simulink model "snap2_f_200msps_64i_4096c_for_8_11_2124fpg"  (R2019a)
settings: Solver=VariableStepDiscrete  StartTime=0.0  StopTime=10.0  RelTol=1e-3
undefined symbols (not defined in the file): datetime, posixtime
libraries: xbsIndex_r4 x17164, casper_library_misc x251, xps_library x14, casper_library_delays x12, casper_library_sources x4
(part 4 of 196)

== system snap2_f_200msps_64i_4096c_for_8_11_2124fpg/delay ==
  sync : Inport
  din : Inport  Port=2
  0 : Subsystem
  1 : Subsystem
  10 : Subsystem
  11 : Subsystem
  12 : Subsystem
  13 : Subsystem
  14 : Subsystem
  15 : Subsystem
  16 : Subsystem
  17 : Subsystem
  18 : Subsystem
  19 : Subsystem
  2 : Subsystem
  20 : Subsystem
  21 : Subsystem
  22 : Subsystem
  23 : Subsystem
  24 : Subsystem
  25 : Subsystem
  26 : Subsystem
  27 : Subsystem
  28 : Subsystem
  29 : Subsystem
  3 : Subsystem
  30 : Subsystem
  31 : Subsystem
  32 : Subsystem
  33 : Subsystem
  34 : Subsystem
  35 : Subsystem
  36 : Subsystem
  37 : Subsystem
  38 : Subsystem
  39 : Subsystem
  4 : Subsystem
  40 : Subsystem
  41 : Subsystem
  42 : Subsystem
  43 : Subsystem
  44 : Subsystem
  45 : Subsystem
  46 : Subsystem
  47 : Subsystem
  48 : Subsystem
  49 : Subsystem
  5 : Subsystem
  50 : Subsystem
  51 : Subsystem
  52 : Subsystem
  53 : Subsystem
  54 : Subsystem
  55 : Subsystem
  56 : Subsystem
  57 : Subsystem
  58 : Subsystem
  59 : Subsystem
  6 : Subsystem
  60 : Subsystem
  61 : Subsystem
  62 : Subsystem
  63 : Subsystem
  7 : Subsystem
  8 : Subsystem
  9 : Subsystem
  Constant : xbsIndex_r4/Constant  const=2^max_delay_bits  gui_display_data_type=Fixed-point  arith_type=Unsigned  n_bits=32  bin_pt=0  preci_type=Single  exp_width=8  frac_width=24  explicit_period=on  period=1  equ=P=C  opselect=C  inp2=PCIN>>17  opr=+  inp1=P  carry=CIN  dbl_ovrd=off  block_type=constant
  Delay : xbsIndex_r4/Delay  rst=off  infoeditControl=Selection of Reset will increase slice count due to use of real FFs and instead of SRLs  en=off  latency=7  reg_retiming=on  dbl_ovrd=off  block_type=delay
  bus_create : Subsystem  AncestorBlock=casper_library_flow_control/bus_create  mask(bus_create){inputNum=64}
      mask prompts: inputNum "Number of inputs:"
      mask init:
      | bus_create_init(gcb,...
      |     'inputNum', inputNum);
  bus_expand : Subsystem  AncestorBlock=casper_library_flow_control/bus_expand  mask(bus_expand){mode=divisions of equal size, outputNum=8*8, outputWidth=10, outputBinaryPt=0, outputArithmeticType=0, show_format=off, outputToWorkspace=off, variablePrefix=out, outputToModelAsWell=on}
      mask prompts: outputNum "Number of outputs:"; outputWidth "Output width:"; outputBinaryPt "Output binary point position:"; outputArithmeticType "Output arithmetic type (ufix=0, fix=1, bool=2):"; show_format "Print format string?"; outputToWorkspace "Output to workspace?"; variablePrefix "Variable name prefix:"; outputToModelAsWell "Output to model as well?"
      mask help:
      | Expands a 'bus' made using a bus_create or Xilinx
      | concat block.
      | The input will be divided into the specified number
      | of outputs with the specified arithmetic types.
      | Divisions are made from most significant bit.
      mask init: same as snap2_f_200msps_64i_4096c_for_8_11_2124fpg/autocorr/bus_expand
      settings: AttributesFormatString=64 outputs
      mask TypeOptions mode: divisions of equal size | divisions of arbitrary size
      mask Callback mode: bus_expand_callback();
      mask Callback outputWidth: bus_expand_callback();
      mask Callback outputToWorkspace: bus_expand_callback();
  max_delay : Subsystem  Tag=xps:sw_reg  AncestorBlock=xps_library/Memory/software_register  mask(swreg){io_dir=To Processor, io_delay=0, init_val=0, sample_period=1, names=reg, bitwidths=32, bin_pts=0, arith_types=0, sim_port=on, show_format=off, desc_str=|}
      mask desc_str=|
      | Interpretted as a unint32 value, contains the maximum
      | allowed delay, in ADC samples, which may be applied
      | to a data stream.
      mask prompts: io_dir "I/O direction"; io_delay "I/O delay"; init_val "Initial Value"; sample_period "Sample period"; names "Bitfield names [msb...lsb]"; bitwidths "Bitfield widths"; bin_pts "Bitfield binary pts"; arith_types "Bitfield types, ufix=0, fix=1, bool=2"; sim_port "Provide sim input/output?"; show_format "Print format string?"; desc_str "Description"
      mask help: A 32-bit software-accessible register. Can be divided into bitfields of varying widths and types using the fields in the block mask.
      mask init:
      | swreg_init(gcb)
      settings: AttributesFormatString=1output
      mask TypeOptions io_dir: To Processor | From Processor
      mask Callback io_dir:
      | mask_enables = get_param(gcb, 'MaskEnables');
      | mask_names = get_param(gcb, 'MaskNames');
      | if strcmp(get_param(gcb, 'io_dir'), 'To Processor'),
      |     mask_enables{ismember(mask_names, 'sample_period')} = 'off';
      | else
      |     mask_enables{ismember(mask_names, 'sample_period')} = 'on';
      | end
      | set_param(gcb, 'MaskEnables', mask_enables);
      mask DialogControl ParameterTabVar0 Prompt: Setup
  sync_out : Outport
  dout : Outport  Port=2
  wires:
  sync/1 -> Delay/1
  din/1 -> bus_expand/1(bus_in)
  bus_expand/1(msb_out64) -> 0/1(din)
  bus_create/1(bus_out) -> dout/1
  Constant/1 -> max_delay/1(out_reg)
  Delay/1 -> sync_out/1
  1/1(dout) -> bus_create/2(in2)
  0/1(dout) -> bus_create/1(in1)
  bus_expand/2(out63) -> 1/1(din)
  bus_expand/19(out46) -> 18/1(din)
  bus_expand/21(out44) -> 20/1(din)
  bus_expand/23(out42) -> 22/1(din)
  bus_expand/29(out36) -> 28/1(din)
  bus_expand/31(out34) -> 30/1(din)
  bus_expand/33(out32) -> 32/1(din)
  bus_expand/37(out28) -> 36/1(din)
  bus_expand/41(out24) -> 40/1(din)
  bus_expand/43(out22) -> 42/1(din)
  bus_expand/45(out20) -> 44/1(din)
  bus_expand/47(out18) -> 46/1(din)
  bus_expand/51(out14) -> 50/1(din)
  bus_expand/55(out10) -> 54/1(din)
  bus_expand/59(out6) -> 58/1(din)
  bus_expand/61(out4) -> 60/1(din)
  bus_expand/63(out2) -> 62/1(din)
  17/1(dout) -> bus_create/18(in18)
  3/1(dout) -> bus_create/4(in4)
  5/1(dout) -> bus_create/6(in6)
  7/1(dout) -> bus_create/8(in8)
  9/1(dout) -> bus_create/10(in10)
  11/1(dout) -> bus_create/12(in12)
  13/1(dout) -> bus_create/14(in14)
  15/1(dout) -> bus_create/16(in16)
  19/1(dout) -> bus_create/20(in20)
  21/1(dout) -> bus_create/22(in22)
  23/1(dout) -> bus_create/24(in24)
  25/1(dout) -> bus_create/26(in26)
  27/1(dout) -> bus_create/28(in28)
  29/1(dout) -> bus_create/30(in30)
  31/1(dout) -> bus_create/32(in32)
  33/1(dout) -> bus_create/34(in34)
  35/1(dout) -> bus_create/36(in36)
  37/1(dout) -> bus_create/38(in38)
  39/1(dout) -> bus_create/40(in40)
  41/1(dout) -> bus_create/42(in42)
  43/1(dout) -> bus_create/44(in44)
  45/1(dout) -> bus_create/46(in46)
  47/1(dout) -> bus_create/48(in48)
  49/1(dout) -> bus_create/50(in50)
  51/1(dout) -> bus_create/52(in52)
  53/1(dout) -> bus_create/54(in54)
  55/1(dout) -> bus_create/56(in56)
  57/1(dout) -> bus_create/58(in58)
  59/1(dout) -> bus_create/60(in60)
  61/1(dout) -> bus_create/62(in62)
  63/1(dout) -> bus_create/64(in64)
  16/1(dout) -> bus_create/17(in17)
  2/1(dout) -> bus_create/3(in3)
  4/1(dout) -> bus_create/5(in5)
  6/1(dout) -> bus_create/7(in7)
  8/1(dout) -> bus_create/9(in9)
  10/1(dout) -> bus_create/11(in11)
  12/1(dout) -> bus_create/13(in13)
  14/1(dout) -> bus_create/15(in15)
  18/1(dout) -> bus_create/19(in19)
  20/1(dout) -> bus_create/21(in21)
  22/1(dout) -> bus_create/23(in23)
  24/1(dout) -> bus_create/25(in25)
  26/1(dout) -> bus_create/27(in27)
  28/1(dout) -> bus_create/29(in29)
  30/1(dout) -> bus_create/31(in31)
  32/1(dout) -> bus_create/33(in33)
  34/1(dout) -> bus_create/35(in35)
  36/1(dout) -> bus_create/37(in37)
  38/1(dout) -> bus_create/39(in39)
  40/1(dout) -> bus_create/41(in41)
  42/1(dout) -> bus_create/43(in43)
  44/1(dout) -> bus_create/45(in45)
  46/1(dout) -> bus_create/47(in47)
  48/1(dout) -> bus_create/49(in49)
  50/1(dout) -> bus_create/51(in51)
  52/1(dout) -> bus_create/53(in53)
  54/1(dout) -> bus_create/55(in55)
  56/1(dout) -> bus_create/57(in57)
  58/1(dout) -> bus_create/59(in59)
  60/1(dout) -> bus_create/61(in61)
  62/1(dout) -> bus_create/63(in63)
  bus_expand/3(out62) -> 2/1(din)
  bus_expand/5(out60) -> 4/1(din)
  bus_expand/7(out58) -> 6/1(din)
  bus_expand/9(out56) -> 8/1(din)
  bus_expand/11(out54) -> 10/1(din)
  bus_expand/13(out52) -> 12/1(din)
  bus_expand/15(out50) -> 14/1(din)
  bus_expand/25(out40) -> 24/1(din)
  bus_expand/27(out38) -> 26/1(din)
  bus_expand/35(out30) -> 34/1(din)
  bus_expand/39(out26) -> 38/1(din)
  bus_expand/49(out16) -> 48/1(din)
  bus_expand/53(out12) -> 52/1(din)
  bus_expand/57(out8) -> 56/1(din)
  bus_expand/17(out48) -> 16/1(din)
  bus_expand/4(out61) -> 3/1(din)
  bus_expand/6(out59) -> 5/1(din)
  bus_expand/8(out57) -> 7/1(din)
  bus_expand/10(out55) -> 9/1(din)
  bus_expand/12(out53) -> 11/1(din)
  bus_expand/14(out51) -> 13/1(din)
  bus_expand/16(out49) -> 15/1(din)
  bus_expand/20(out45) -> 19/1(din)
  bus_expand/22(out43) -> 21/1(din)
  bus_expand/24(out41) -> 23/1(din)
  bus_expand/26(out39) -> 25/1(din)
  bus_expand/28(out37) -> 27/1(din)
  bus_expand/30(out35) -> 29/1(din)
  bus_expand/32(out33) -> 31/1(din)
  bus_expand/34(out31) -> 33/1(din)
  bus_expand/36(out29) -> 35/1(din)
  bus_expand/38(out27) -> 37/1(din)
  bus_expand/40(out25) -> 39/1(din)
  bus_expand/42(out23) -> 41/1(din)
  bus_expand/44(out21) -> 43/1(din)
  bus_expand/46(out19) -> 45/1(din)
  bus_expand/48(out17) -> 47/1(din)
  bus_expand/50(out15) -> 49/1(din)
  bus_expand/52(out13) -> 51/1(din)
  bus_expand/54(out11) -> 53/1(din)
  bus_expand/56(out9) -> 55/1(din)
  bus_expand/58(out7) -> 57/1(din)
  bus_expand/60(out5) -> 59/1(din)
  bus_expand/62(out3) -> 61/1(din)
  bus_expand/64(lsb_out1) -> 63/1(din)
  bus_expand/18(out47) -> 17/1(din)

== system snap2_f_200msps_64i_4096c_for_8_11_2124fpg/eq ==
  sync : Inport
  din : Inport  Port=2
  bus_create2 : Subsystem  AncestorBlock=casper_library_flow_control/bus_create  mask(bus_create){inputNum=4}
      mask prompts: inputNum "Number of inputs:"
      mask init:
      | bus_create_init(gcb,...
      |     'inputNum', inputNum);
  bus_expand4 : Subsystem  AncestorBlock=casper_library_flow_control/bus_expand  mask(bus_expand){mode=divisions of equal size, outputNum=4, outputWidth=36*8, outputBinaryPt=0, outputArithmeticType=0, show_format=off, outputToWorkspace=off, variablePrefix=out, outputToModelAsWell=on}
      mask prompts: outputNum "Number of outputs:"; outputWidth "Output width:"; outputBinaryPt "Output binary point position:"; outputArithmeticType "Output arithmetic type (ufix=0, fix=1, bool=2):"; show_format "Print format string?"; outputToWorkspace "Output to workspace?"; variablePrefix "Variable name prefix:"; outputToModelAsWell "Output to model as well?"
      mask help:
      | Expands a 'bus' made using a bus_create or Xilinx
      | concat block.
      | The input will be divided into the specified number
      | of outputs with the specified arithmetic types.
      | Divisions are made from most significant bit.
      mask init: same as snap2_f_200msps_64i_4096c_for_8_11_2124fpg/autocorr/bus_expand
      settings: AttributesFormatString=4 outputs
      mask TypeOptions mode: divisions of equal size | divisions of arbitrary size
      mask Callback mode: bus_expand_callback();
      mask Callback outputWidth: bus_expand_callback();
      mask Callback outputToWorkspace: bus_expand_callback();
  core0 : Subsystem  mask{nchan=12-3, nants=16}
      mask prompts: nchan "Number of fft channels (2^?)"; nants "Number of Antennas"
  core1 : Subsystem  mask{nchan=12-3, nants=16}
      mask prompts: nchan "Number of fft channels (2^?)"; nants "Number of Antennas"
  core2 : Subsystem  mask{nchan=12-3, nants=16}
      mask prompts: nchan "Number of fft channels (2^?)"; nants "Number of Antennas"
  core3 : Subsystem  mask{nchan=12-3, nants=16}
      mask prompts: nchan "Number of fft channels (2^?)"; nants "Number of Antennas"
  sync_out : Outport
  dout : Outport  Port=2
  wires:
  core0/1(sync_out) -> sync_out/1
  din/1 -> bus_expand4/1(bus_in)
  sync/1 -> [sync_in]
  [sync_in] -> core0/1(sync)
  [sync_in] -> core1/1(sync)
  [sync_in] -> core2/1(sync)
  [sync_in] -> core3/1(sync)
  bus_expand4/1(msb_out4) -> core0/2(in)
  bus_expand4/2(out3) -> core1/2(in)
  bus_expand4/3(out2) -> core2/2(in)
  bus_expand4/4(lsb_out1) -> core3/2(in)
  core1/1(sync_out) -> ?
  core2/1(sync_out) -> ?
  core3/1(sync_out) -> ?
  core1/2(dout) -> bus_create2/2(in2)
  core2/2(dout) -> bus_create2/3(in3)
  core0/2(dout) -> bus_create2/1(in1)
  core3/2(dout) -> bus_create2/4(in4)
  bus_create2/1(bus_out) -> dout/1

== system snap2_f_200msps_64i_4096c_for_8_11_2124fpg/eth ==
  note: bit 19
  note: bit 18
  note: bit 1
  note: bit 0
  note: only allow enable
      | state to change at the end
      | of a packet.
      |
      | This avoids overflows arising
      | from part of a packet being
      | clocked in to the core
      | but the EOF being disable
  data : Inport
  vld : Inport  Port=2
  eof : Inport  Port=3
  dest_ip : Inport  Port=4
  port : Inport  Port=5
  Constant : xbsIndex_r4/Constant  const=0  gui_display_data_type=Boolean  arith_type=Boolean  n_bits=1  bin_pt=0  preci_type=Single  exp_width=8  frac_width=24  explicit_period=on  period=1  equ=P=C  opselect=C  inp2=PCIN>>17  opr=+  inp1=P  carry=CIN  dbl_ovrd=off  block_type=constant
  Constant1 : xbsIndex_r4/Constant  const=0  gui_display_data_type=Boolean  arith_type=Boolean  n_bits=1  bin_pt=0  preci_type=Single  exp_width=8  frac_width=24  explicit_period=on  period=1  equ=P=C  opselect=C  inp2=PCIN>>17  opr=+  inp1=P  carry=CIN  dbl_ovrd=off  block_type=constant
  Delay{,1..4} (5 alike) : xbsIndex_r4/Delay  rst=off  infoeditControl=Selection of Reset will increase slice count due to use of real FFs and instead of SRLs  en=off  latency=1  reg_retiming=off  dbl_ovrd=off  block_type=delay
  Logical : xbsIndex_r4/Logical  logical_function=AND  inputs=2  en=off  latency=0  precision=Full  arith_type=Unsigned  n_bits=16  bin_pt=0  align_bp=on  dbl_ovrd=off  block_type=logical
  Logical1 : xbsIndex_r4/Logical  logical_function=OR  inputs=2  en=off  latency=0  precision=Full  arith_type=Unsigned  n_bits=16  bin_pt=0  align_bp=on  dbl_ovrd=off  block_type=logical
  Register : xbsIndex_r4/Register  init=0  rst=off  en=on  dbl_ovrd=off  block_type=register
  Slice : xbsIndex_r4/Slice  nbits=1  boolean_output=on  mode=Lower Bit Location + Width  bit1=0  base1=MSB of Input  bit0=0  base0=LSB of Input  dbl_ovrd=off  block_type=slice
  Slice1 : xbsIndex_r4/Slice  nbits=1  boolean_output=on  mode=Lower Bit Location + Width  bit1=0  base1=MSB of Input  bit0=1  base0=LSB of Input  dbl_ovrd=off  block_type=slice
  Slice3 : xbsIndex_r4/Slice  nbits=1  boolean_output=on  mode=Lower Bit Location + Width  bit1=0  base1=MSB of Input  bit0=2+16  base0=LSB of Input  dbl_ovrd=off  block_type=slice
  Slice4 : xbsIndex_r4/Slice  nbits=1  boolean_output=on  mode=Lower Bit Location + Width  bit1=0  base1=MSB of Input  bit0=3+16  base0=LSB of Input  dbl_ovrd=off  block_type=slice
  ctrl : Subsystem  Tag=xps:sw_reg  AncestorBlock=xps_library/Memory/software_register  mask(swreg){io_dir=From Processor, io_delay=0, init_val=0, sample_period=1, names=reg, bitwidths=32, bin_pts=0, arith_types=0, sim_port=on, show_format=off, desc_str=|}
      mask desc_str=|
      | 40GbE control register. Bit ``0`` is an active high reset
      | of the 40GbE interface core. Bit ``1`` is an active
      | high transmission enable for the core, which takes
      | effect either after the next packet is sent, or when
      | the ``force`` flag is asserted. Bit ``18`` is
      | an active high reset for statistics provided by the
      | Ethernet core. Bit ``19`` is an active high ``force``
      | signal, which causes the current transmission enable
      | flag to immediately take effect.
      mask prompts: io_dir "I/O direction"; io_delay "I/O delay"; init_val "Initial Value"; sample_period "Sample period"; names "Bitfield names [msb...lsb]"; bitwidths "Bitfield widths"; bin_pts "Bitfield binary pts"; arith_types "Bitfield types, ufix=0, fix=1, bool=2"; sim_port "Provide sim input/output?"; show_format "Print format string?"; desc_str "Description"
      mask help: A 32-bit software-accessible register. Can be divided into bitfields of varying widths and types using the fields in the block mask.
      mask init:
      | swreg_init(gcb)
      settings: AttributesFormatString=1input
      mask TypeOptions io_dir: To Processor | From Processor
      mask Callback io_dir:
      | mask_enables = get_param(gcb, 'MaskEnables');
      | mask_names = get_param(gcb, 'MaskNames');
      | if strcmp(get_param(gcb, 'io_dir'), 'To Processor'),
      |     mask_enables{ismember(mask_names, 'sample_period')} = 'off';
      | else
      |     mask_enables{ismember(mask_names, 'sample_period')} = 'on';
      | end
      | set_param(gcb, 'MaskEnables', mask_enables);
      mask DialogControl ParameterTabVar0 Prompt: Setup
  forty_gbe : Subsystem  Tag=xps:forty_gbe  AncestorBlock=xps_library/IO/forty_gbe  InitFcn=forty_gbe_init  mask(forty_gbe){flavour=cx4, slot=1, port_r1=0, port_r2_cx4=0, port_r2_sfpp=0, port=0, rx_dist_ram=on, large_frames=on, input_pipeline_delay=0, show_param=on, pre_emph_r2=0.74, pre_emph=3, post_emph_r2=0.18, rxeqmix_r2=7, swing_r2=940, swing=800, fab_en=on, fab_mac=hex2dec('123456780000'), fab_ip=192*(2^24) + 168*(2^16) + 5*(2^8) + 20*(2^0), fab_udp=10000, fab_gate=1, cpu_rx_en=off, cpu_tx_en=off, ttl=hex2dec('FF'), promisc_mode=off, debug_ctr_width=32, debug_en_all=off, debug_dis_all=off, txctr=on, txerrctr=off, txerrctr_len=100, txofctr=on, txfullctr=on, txvldctr=on, txsnaplen=0 - no snap, rxctr=off, rxerrctr=off, rxerrctr_len=100, rxofctr=off, rxbadctr=off, rxvldctr=off, rxeofctr=off, rxsnaplen=0 - no snap, rxsrcip=off, rxdstip=off}
      mask prompts: flavour "Mezzanine card flavour"; slot "Card slot"; port_r1 "Port"; port_r2_cx4 "Port"; port_r2_sfpp "Port"; rx_dist_ram "Shallow RX Fifo (Beware overruns!)"; large_frames "Enable Large TX Frames (8k+512)"; input_pipeline_delay "Incoming pipeline delay"; show_param "---------------------- Show Implementation Parameters -----------------------------------------------------------------------------------"; pre_emph_r2 "Pre-emphasis (dB)"; pre_emph "Pre-emphasis"; post_emph_r2 "Post-emphasis (dB)"; rxeqmix_r2 "Receive equalisation"; swing_r2 "Driver Swing Control (mVppd)"; swing "Differential swing"; fab_en "Enable fabric on startup"; fab_mac "Fabric MAC Address"; fab_ip "Fabric IP Address"; fab_udp "Fabric UDP Port"; fab_gate "Fabric Gateway"; cpu_rx_en "Enable CPU RX"; cpu_tx_en "Enable CPU TX"; promisc_mode "Promiscuous mode"; debug_ctr_width "Debug counter width"; debug_en_all "Enable all counters (overrides individual settings)"; debug_dis_all "Disable all counters (overrides individual settings)"; txctr "TX - core_txctr"; txerrctr "TX packet length error - core_txerrctr"; txerrctr_len "-- expected TX packet length"; txofctr "TX overflow - core_txofctr"; txfullctr "TX full - core_txfullctr"; txvldctr "TX valid cycle - core_txvldctr"; txsnaplen "TX snapshot length"; rxctr "RX - core_rxctr"; rxerrctr "RX packet length error - core_rxerrctr"; rxerrctr_len "-- expected RX packet length"; rxofctr "RX overflow - core_rxofctr"; rxbadctr "RX bad - core_rxbadctr"; rxvldctr "RX valid cycle - core_rxvldctr"; rxeofctr "RX eof - core_rxeofctr"; rxsnaplen "RX snapshot length"; rxsrcip "RX snapshot - include source IPs"; rxdstip "RX snapshot - include destination IPs"
      mask help: This block sends and receives UDP frames (packets). It accepts a 256 bit wide data stream with user-determined frame breaks. The data stream is wrapped in a UDP frame for transmission. Incoming UDP packets are unwrapped and the data presented as a 256 bit wide stream.
      mask init:
      | temp = eval(get_param(gcb, 'debug_ctr_width'));
      | if temp <= 0,
      |     error('Debug counters cannot be <= zero.');
      | end
      | forty_gbe_mask(gcb)
      settings: AttributesFormatString=incoming_latency=0
      mask TypeOptions flavour: sfp+ | cx4
      mask Callback flavour: forty_gbe_callback
      mask TypeOptions slot: 0 | 1
      mask TypeOptions port_r1: 0 | 1 | 2 | 3
      mask TypeOptions port_r2_cx4: 0 | 1 | 2
      mask TypeOptions port_r2_sfpp: 0 | 1 | 2 | 3
      mask TypeOptions port: 0 | 1 | 2 | 3
      mask Callback show_param: forty_gbe_callback
      mask TypeOptions pre_emph_r2: 0.15 | 0.3 | 0.45 | 0.61 | 0.74 | 0.91 | 1.07 | 1.25 | 1.36 | 1.55 | 1.74 | 1.94 | 2.11 | 2.32 | 2.54 | 2.77
      mask TypeOptions pre_emph: 0 | 1 | 2 | 3
      mask TypeOptions post_emph_r2: 0.18 | 0.19 | 0.2 | 0.39 | 0.63 | 0.82 | 1.07 | 1.32 | 1.6 | 1.65 | 1.94 | 2.21 | 2.52 | 2.76 | 3.08 | 3.41 | 3.77 | 3.97 | 4.36 | 4.73 | 5.16 | 5.47 | 5.93 | 6.38 | 6.89
      mask TypeOptions rxeqmix_r2: 0 | 1 | 2 | 3 | 4 | 5 | 6 | 7
      mask TypeOptions swing_r2: 110 | 210 | 310 | 400 | 480 | 570 | 660 | 740 | 810 | 880 | 940 | 990 | 1040 | 1080 | 1110 | 1130
      mask TypeOptions swing: 400 | 500 | 600 | 700 | 800
      mask Callback debug_en_all:
      | if strcmp(get_param(gcb, 'debug_en_all'), 'on')
      |     set_param(gcb, 'debug_dis_all', 'off')
      | end
      mask Callback debug_dis_all:
      | if strcmp(get_param(gcb, 'debug_dis_all'), 'on')
      |     set_param(gcb, 'debug_en_all', 'off')
      | end
      mask Callback txerrctr:
      | blk = gcb;
      | mask_visibilities = get_param(blk, 'MaskVisibilities');
      | mask_names = get_param(blk, 'MaskNames');
      | if strcmp(get_param(blk, 'txerrctr'), 'on')
      |     mask_visibilities{ismember(mask_names, 'txerrctr_len')} = 'on';
      | else
      |     mask_visibilities{ismember(mask_names, 'txerrctr_len')} = 'off';
      | end
      | set_param(blk, 'MaskVisibilities', mask_visibilities);
      mask TypeOptions txsnaplen: 0 - no snap | 256 | 512 | 1024 | 2048 | 4096 | 8192 | 16384
      mask Callback rxerrctr:
      | blk = gcb;
      | mask_visibilities = get_param(blk, 'MaskVisibilities');
      | mask_names = get_param(blk, 'MaskNames');
      | if strcmp(get_param(blk, 'rxerrctr'), 'on')
      |     mask_visibilities{ismember(mask_names, 'rxerrctr_len')} = 'on';
      | else
      |     mask_visibilities{ismember(mask_names, 'rxerrctr_len')} = 'off';
      | end
      | set_param(blk, 'MaskVisibilities', mask_visibilities);
      mask TypeOptions rxsnaplen: 0 - no snap | 256 | 512 | 1024 | 2048 | 4096 | 8192 | 16384
      mask DialogControl ParameterTabVar0 Prompt: Core
      mask DialogControl ParameterTabVar01 Prompt: Debug counters
      mask DialogControl Control2 Prompt: -------------------------------------
      mask DialogControl Control2_copy Prompt: -------------------------------------
  wires:
  port/1 -> Delay3/1
  forty_gbe/12(rx_overrun) -> [gbe_sw_rx_orun]
  [gbe_sw_rst] -> forty_gbe/1(rst)
  data/1 -> Delay/1
  eof/1 -> Logical1/2, Delay4/1
  dest_ip/1 -> Delay2/1
  Logical/1 -> Delay1/1
  vld/1 -> Logical/1
  ctrl/1(in_reg) -> Slice4/1, Slice3/1, Slice1/1, Slice/1
  Slice/1 -> [gbe_sw_rst]
  Slice1/1 -> [gbe_sw_en]
  Slice3/1 -> [gbe_stat_rst]
  [gbe_stat_rst] -> forty_gbe/9(debug_rst)
  forty_gbe/5(tx_overflow) -> [gbe_sw_tx_oflow]
  forty_gbe/4(tx_afull) -> [gbe_sw_tx_afull]
  forty_gbe/3(led_tx) -> [gbe_sw_led_tx]
  forty_gbe/2(led_rx) -> [gbe_sw_led_rx]
  forty_gbe/1(led_up) -> [gbe_sw_led_up]
  forty_gbe/11(rx_bad_frame) -> [gbe_sw_rx_bfr]
  Constant/1 -> forty_gbe/7(rx_ack)
  [gbe_sw_en] -> Register/1
  Register/1 -> Logical/2
  Slice4/1 -> [force_en]
  Logical1/1 -> Register/2
  [force_en] -> Logical1/1
  Delay/1 -> forty_gbe/2(tx_data)
  Delay1/1 -> forty_gbe/3(tx_valid)
  Delay2/1 -> forty_gbe/4(tx_dest_ip)
  Delay3/1 -> forty_gbe/5(tx_dest_port)
  Delay4/1 -> forty_gbe/6(tx_end_of_frame)
  Constant1/1 -> forty_gbe/8(rx_overrun_ack)

== system snap2_f_200msps_64i_4096c_for_8_11_2124fpg/input ==
  adc : Inport
  noise : Inport  Port=2
  sync : Inport  Port=3
  Constant4 : xbsIndex_r4/Constant  const=0  gui_display_data_type=Fixed-point  arith_type=Unsigned  n_bits=10*64  bin_pt=0  preci_type=Single  exp_width=8  frac_width=24  explicit_period=on  period=1  equ=P=C  opselect=C  inp2=PCIN>>17  opr=+  inp1=P  carry=CIN  dbl_ovrd=off  block_type=constant
  bit_stats : Subsystem
  bus_create2 : Subsystem  AncestorBlock=casper_library_flow_control/bus_create  mask(bus_create){inputNum=4}
      mask prompts: inputNum "Number of inputs:"
      mask init:
      | bus_create_init(gcb,...
      |     'inputNum', inputNum);
  bus_mux : Subsystem  AncestorBlock=casper_library_bus/bus_mux  mask(bus_mux){n_inputs=3, n_bits=10 * ones(64,1), cmplx=off, misc=on, mux_latency=1}
      mask prompts: n_inputs "number of inputs"; n_bits "input bit widths"; cmplx "complex data"; misc "misc support"; mux_latency "mux latency"
      mask help: Mux components of bus
      mask init:
      | bus_mux_init(gcb, ...
      |     'n_inputs', n_inputs, ...
      |     'n_bits', n_bits, ...
      |     'cmplx', cmplx, ...
      |     'misc', misc, ...
      |     'mux_latency', mux_latency);
  rms : Subsystem
  slice3 : xbsIndex_r4/Slice  nbits=2*64  boolean_output=off  mode=Lower Bit Location + Width  bit1=0  base1=MSB of Input  bit0=0  base0=LSB of Input  dbl_ovrd=off  block_type=slice
  source_sel0 : Subsystem  Tag=xps:sw_reg  AncestorBlock=xps_library/Memory/software_register  mask(swreg){io_dir=From Processor, io_delay=0, init_val=0, sample_period=1, names=reg, bitwidths=32, bin_pts=0, arith_types=0, sim_port=on, show_format=off, desc_str=|}
      mask desc_str=|
      | Selector control to determine if signal streams carry
      | ADC samples, digital noise samples, or zeros.
      | Streams ``n=0..15`` is controlled by register
      | bits ``[2n+1:2n]``. Value ``0`` selects noise;
      | ``1`` selects ADC; ``2`` selects zeros.
      mask prompts: io_dir "I/O direction"; io_delay "I/O delay"; init_val "Initial Value"; sample_period "Sample period"; names "Bitfield names [msb...lsb]"; bitwidths "Bitfield widths"; bin_pts "Bitfield binary pts"; arith_types "Bitfield types, ufix=0, fix=1, bool=2"; sim_port "Provide sim input/output?"; show_format "Print format string?"; desc_str "Description"
      mask help: A 32-bit software-accessible register. Can be divided into bitfields of varying widths and types using the fields in the block mask.
      mask init:
      | swreg_init(gcb)
      settings: AttributesFormatString=1input
      mask TypeOptions io_dir: To Processor | From Processor
      mask Callback io_dir:
      | mask_enables = get_param(gcb, 'MaskEnables');
      | mask_names = get_param(gcb, 'MaskNames');
      | if strcmp(get_param(gcb, 'io_dir'), 'To Processor'),
      |     mask_enables{ismember(mask_names, 'sample_period')} = 'off';
      | else
      |     mask_enables{ismember(mask_names, 'sample_period')} = 'on';
      | end
      | set_param(gcb, 'MaskEnables', mask_enables);
      mask DialogControl ParameterTabVar0 Prompt: Setup
  source_sel1 : Subsystem  Tag=xps:sw_reg  AncestorBlock=xps_library/Memory/software_register  mask(swreg){io_dir=From Processor, io_delay=0, init_val=0, sample_period=1, names=reg, bitwidths=32, bin_pts=0, arith_types=0, sim_port=on, show_format=off, desc_str=|}
      mask desc_str=|
      | Selector control to determine if signal streams carry
      | ADC samples, digital noise samples, or zeros.
      | Streams ``n=16..31`` is controlled by register
      | bits ``[2(n-16)+1:2(n-16)]``. Value ``0`` selects noise;
      | ``1`` selects ADC; ``2`` selects zeros.
      mask prompts: io_dir "I/O direction"; io_delay "I/O delay"; init_val "Initial Value"; sample_period "Sample period"; names "Bitfield names [msb...lsb]"; bitwidths "Bitfield widths"; bin_pts "Bitfield binary pts"; arith_types "Bitfield types, ufix=0, fix=1, bool=2"; sim_port "Provide sim input/output?"; show_format "Print format string?"; desc_str "Description"
      mask help: A 32-bit software-accessible register. Can be divided into bitfields of varying widths and types using the fields in the block mask.
      mask init:
      | swreg_init(gcb)
      settings: AttributesFormatString=1input
      mask TypeOptions io_dir: To Processor | From Processor
      mask Callback io_dir:
      | mask_enables = get_param(gcb, 'MaskEnables');
      | mask_names = get_param(gcb, 'MaskNames');
      | if strcmp(get_param(gcb, 'io_dir'), 'To Processor'),
      |     mask_enables{ismember(mask_names, 'sample_period')} = 'off';
      | else
      |     mask_enables{ismember(mask_names, 'sample_period')} = 'on';
      | end
      | set_param(gcb, 'MaskEnables', mask_enables);
      mask DialogControl ParameterTabVar0 Prompt: Setup
  source_sel2 : Subsystem  Tag=xps:sw_reg  AncestorBlock=xps_library/Memory/software_register  mask(swreg){io_dir=From Processor, io_delay=0, init_val=0, sample_period=1, names=reg, bitwidths=32, bin_pts=0, arith_types=0, sim_port=on, show_format=off, desc_str=|}
      mask desc_str=|
      | Selector control to determine if signal streams carry
      | ADC samples, digital noise samples, or zeros.
      | Streams ``n=32..47`` is controlled by register
      | bits ``[2(n-32)+1:2(n-32)]``. Value ``0`` selects noise;
      | ``1`` selects ADC; ``2`` selects zeros.
      mask prompts: io_dir "I/O direction"; io_delay "I/O delay"; init_val "Initial Value"; sample_period "Sample period"; names "Bitfield names [msb...lsb]"; bitwidths "Bitfield widths"; bin_pts "Bitfield binary pts"; arith_types "Bitfield types, ufix=0, fix=1, bool=2"; sim_port "Provide sim input/output?"; show_format "Print format string?"; desc_str "Description"
      mask help: A 32-bit software-accessible register. Can be divided into bitfields of varying widths and types using the fields in the block mask.
      mask init:
      | swreg_init(gcb)
      settings: AttributesFormatString=1input
      mask TypeOptions io_dir: To Processor | From Processor
      mask Callback io_dir:
      | mask_enables = get_param(gcb, 'MaskEnables');
      | mask_names = get_param(gcb, 'MaskNames');
      | if strcmp(get_param(gcb, 'io_dir'), 'To Processor'),
      |     mask_enables{ismember(mask_names, 'sample_period')} = 'off';
      | else
      |     mask_enables{ismember(mask_names, 'sample_period')} = 'on';
      | end
      | set_param(gcb, 'MaskEnables', mask_enables);
      mask DialogControl ParameterTabVar0 Prompt: Setup
  source_sel3 : Subsystem  Tag=xps:sw_reg  AncestorBlock=xps_library/Memory/software_register  mask(swreg){io_dir=From Processor, io_delay=0, init_val=0, sample_period=1, names=reg, bitwidths=32, bin_pts=0, arith_types=0, sim_port=on, show_format=off, desc_str=|}
      mask desc_str=|
      | Selector control to determine if signal streams carry
      | ADC samples, digital noise samples, or zeros.
      | Streams ``n=48..63`` is controlled by register
      | bits ``[2(n-48)+1:2(n-48)]``. Value ``0`` selects noise;
      | ``1`` selects ADC; ``2`` selects zeros.
      mask prompts: io_dir "I/O direction"; io_delay "I/O delay"; init_val "Initial Value"; sample_period "Sample period"; names "Bitfield names [msb...lsb]"; bitwidths "Bitfield widths"; bin_pts "Bitfield binary pts"; arith_types "Bitfield types, ufix=0, fix=1, bool=2"; sim_port "Provide sim input/output?"; show_format "Print format string?"; desc_str "Description"
      mask help: A 32-bit software-accessible register. Can be divided into bitfields of varying widths and types using the fields in the block mask.
      mask init:
      | swreg_init(gcb)
      settings: AttributesFormatString=1input
      mask TypeOptions io_dir: To Processor | From Processor
      mask Callback io_dir:
      | mask_enables = get_param(gcb, 'MaskEnables');
      | mask_names = get_param(gcb, 'MaskNames');
      | if strcmp(get_param(gcb, 'io_dir'), 'To Processor'),
      |     mask_enables{ismember(mask_names, 'sample_period')} = 'off';
      | else
      |     mask_enables{ismember(mask_names, 'sample_period')} = 'on';
      | end
      | set_param(gcb, 'MaskEnables', mask_enables);
      mask DialogControl ParameterTabVar0 Prompt: Setup
  sync_out : Outport
  dout : Outport  Port=2
  wires:
  Constant4/1 -> bus_mux/4(d2)
  sync/1 -> bus_mux/5(misci)
  bus_mux/1(out) -> dout/1, bit_stats/1(din), rms/1(in)
  slice3/1 -> bus_mux/1(sel)
  adc/1 -> bus_mux/3(d1)
  noise/1 -> bus_mux/2(d0)
  bus_mux/2(misco) -> sync_out/1
  source_sel1/1(in_reg) -> bus_create2/2(in2)
  source_sel0/1(in_reg) -> bus_create2/1(in1)
  source_sel2/1(in_reg) -> bus_create2/3(in3)
  bus_create2/1(bus_out) -> slice3/1
  source_sel3/1(in_reg) -> bus_create2/4(in4)

== system snap2_f_200msps_64i_4096c_for_8_11_2124fpg/noise ==
  sync_in : Inport
  arm_noise : Inport  Port=2
  BitBasher : xbsIndex_r4/BitBasher  bitexpr=|  display_expr=off  sr_1=1  arith_type1=Unsigned  bin_pt1=0  sr_2=2  arith_type2=Unsigned  bin_pt2=0  sr_3=3  arith_type3=Unsigned  bin_pt3=0  sr_4=4  arith_type4=Unsigned  bin_pt4=0  dbl_ovrd=off  block_type=bitbasher
      | <!DOCTYPE HTML PUBLIC "-//W3C//DTD HTML 4.0//EN" "http://www.w3.org/TR/REC-html40/strict.dtd">
      | <html><head><meta name="qrichtext" content="1" /><style type="text/css">
      | p, li { white-space: pre-wrap; }
      | </style></head><body style=" font-family:'Sans'; font-size:10pt; font-weight:400; font-style:normal;">
      | <p style=" margin-top:12px; margin-bottom:12px; margin-left:0px; margin-right:0px; -qt-block-indent:0; text-indent:0px;"><span style=" font-family:'MS Shell Dlg'; font-size:8pt;">s0={8'h5c, s32[7:0], s32[0:7], 8'ha3}<br />s1={8'h5c, s32[15:8], s32[8:15], 8'ha3}<br />s2={8'h5c, s32[23:16], s32[16:23], 8'ha3}<br />s3={8'h5c, s32[31:24], s32[24:31], 8'ha3}</span></p></body></html>
  Constant : Constant  Value=0
  Delay{,1..7} (8 alike) : xbsIndex_r4/Delay  rst=off  infoeditControl=Selection of Reset will increase slice count due to use of real FFs and instead of SRLs  en=off  latency=1  reg_retiming=off  dbl_ovrd=off  block_type=delay
  armed_trigger : casper_library_misc/armed_trigger
      settings: SourceProductName=CASPER DSP Blockset
  bus_create1 : Subsystem  AncestorBlock=casper_library_flow_control/bus_create  mask(bus_create){inputNum=8}
      mask prompts: inputNum "Number of inputs:"
      mask init:
      | bus_create_init(gcb,...
      |     'inputNum', inputNum);
  bus_create2 : Subsystem  AncestorBlock=casper_library_flow_control/bus_create  mask(bus_create){inputNum=8}
      mask prompts: inputNum "Number of inputs:"
      mask init:
      | bus_create_init(gcb,...
      |     'inputNum', inputNum);
  bus_create3 : Subsystem  AncestorBlock=casper_library_flow_control/bus_create  mask(bus_create){inputNum=8}
      mask prompts: inputNum "Number of inputs:"
      mask init:
      | bus_create_init(gcb,...
      |     'inputNum', inputNum);
  bus_create4 : Subsystem  AncestorBlock=casper_library_flow_control/bus_create  mask(bus_create){inputNum=8}
      mask prompts: inputNum "Number of inputs:"
      mask init:
      | bus_create_init(gcb,...
      |     'inputNum', inputNum);
  bus_create5 : Subsystem  AncestorBlock=casper_library_flow_control/bus_create  mask(bus_create){inputNum=8}
      mask prompts: inputNum "Number of inputs:"
      mask init:
      | bus_create_init(gcb,...
      |     'inputNum', inputNum);
  bus_create6 : Subsystem  AncestorBlock=casper_library_flow_control/bus_create  mask(bus_create){inputNum=8}
      mask prompts: inputNum "Number of inputs:"
      mask init:
      | bus_create_init(gcb,...
      |     'inputNum', inputNum);
  bus_create7 : Subsystem  AncestorBlock=casper_library_flow_control/bus_create  mask(bus_create){inputNum=8}
      mask prompts: inputNum "Number of inputs:"
      mask init:
      | bus_create_init(gcb,...
      |     'inputNum', inputNum);
  bus_create8 : Subsystem  AncestorBlock=casper_library_flow_control/bus_create  mask(bus_create){inputNum=8}
      mask prompts: inputNum "Number of inputs:"
      mask init:
      | bus_create_init(gcb,...
      |     'inputNum', inputNum);
  bus_create9 : Subsystem  AncestorBlock=casper_library_flow_control/bus_create  mask(bus_create){inputNum=8}
      mask prompts: inputNum "Number of inputs:"
      mask init:
      | bus_create_init(gcb,...
      |     'inputNum', inputNum);
  noise0 : Subsystem
  noise1 : Subsystem
  noise2 : Subsystem
  noise3 : Subsystem
  octal_mux0 : Subsystem
  octal_mux1 : Subsystem
  octal_mux2 : Subsystem
  octal_mux3 : Subsystem
  octal_mux4 : Subsystem
  octal_mux5 : Subsystem
  octal_mux6 : Subsystem
  octal_mux7 : Subsystem
  seeds0 : Subsystem  Tag=xps:sw_reg  AncestorBlock=xps_library/Memory/software_register  mask(swreg){io_dir=From Processor, io_delay=0, init_val=0, sample_period=1, names=reg, bitwidths=32, bin_pts=0, arith_types=0, sim_port=on, show_format=off, desc_str=|}
      mask desc_str=|
      | Noise generator seed values. If the 32-bit value in this
      | register is ``v``, the seed for the first
      | noise generator is (using Verilog syntax)
      | ``{8'h5c, v[7:0], v[0:7], 8'ha3}``.
      | The seed for the second noise generator is
      | ``{8'h5c, v[15:8], v[8:15], 8'ha3}``.
      | The seed for the third noise generator is
      | ``{8'h5c, v[23:16], v[16:23], 8'ha3}``.
      | The seed for the fourth noise generator is
      | ``{8'h5c, v[31:24], v[24:31], 8'ha3}``
      mask prompts: io_dir "I/O direction"; io_delay "I/O delay"; init_val "Initial Value"; sample_period "Sample period"; names "Bitfield names [msb...lsb]"; bitwidths "Bitfield widths"; bin_pts "Bitfield binary pts"; arith_types "Bitfield types, ufix=0, fix=1, bool=2"; sim_port "Provide sim input/output?"; show_format "Print format string?"; desc_str "Description"
      mask help: A 32-bit software-accessible register. Can be divided into bitfields of varying widths and types using the fields in the block mask.
      mask init:
      | swreg_init(gcb)
      settings: AttributesFormatString=1input
      mask TypeOptions io_dir: To Processor | From Processor
      mask Callback io_dir:
      | mask_enables = get_param(gcb, 'MaskEnables');
      | mask_names = get_param(gcb, 'MaskNames');
      | if strcmp(get_param(gcb, 'io_dir'), 'To Processor'),
      |     mask_enables{ismember(mask_names, 'sample_period')} = 'off';
      | else
      |     mask_enables{ismember(mask_names, 'sample_period')} = 'on';
      | end
      | set_param(gcb, 'MaskEnables', mask_enables);
      mask DialogControl ParameterTabVar0 Prompt: Setup
  noise : Outport
  seed_ld : Outport  Port=2
  wires:
  [seed0] -> noise0/1(seed)
  [seed3] -> noise3/1(seed)
  [seed2] -> noise2/1(seed)
  [seed1] -> noise1/1(seed)
  [seed_ld] -> noise3/2(ld)
  [seed_ld] -> noise2/2(ld)
  [seed_ld] -> noise1/2(ld)
  [seed_ld] -> noise0/2(ld)
  bus_create5/1(bus_out) -> noise/1
  noise0/1(n1) -> [noise0]  "n0"
  noise1/2(n2) -> [noise3]  "n1"
  BitBasher/2 -> [seed1]
  seeds0/1(in_reg) -> BitBasher/1
  BitBasher/3 -> [seed2]
  Constant/1 -> seeds0/1(sim_1)
  BitBasher/1 -> [seed0]
  BitBasher/4 -> [seed3]
  sync_in/1 -> armed_trigger/2
  armed_trigger/1 -> [seed_ld], seed_ld/1
  arm_noise/1 -> armed_trigger/1
  noise0/2(n2) -> [noise1]  "n1"
  noise1/1(n1) -> [noise2]  "n0"
  noise2/1(n1) -> [noise4]  "n0"
  noise2/2(n2) -> [noise5]  "n1"
  noise3/1(n1) -> [noise6]  "n0"
  noise3/2(n2) -> [noise7]  "n1"
  [noise0] -> octal_mux0/1(n0)
  [noise1] -> octal_mux0/2(n1)
  [noise2] -> octal_mux0/3(n2)
  [noise3] -> octal_mux0/4(n3)
  [noise4] -> octal_mux0/5(n4)
  [noise5] -> octal_mux0/6(n5)
  [noise6] -> octal_mux0/7(n6)
  [noise7] -> octal_mux0/8(n7)
  octal_mux0/1(out0) -> bus_create1/1(in1)
  octal_mux0/2(out1) -> bus_create1/2(in2)
  octal_mux0/3(out2) -> bus_create1/3(in3)
  octal_mux0/4(out3) -> bus_create1/4(in4)
  octal_mux0/5(out4) -> bus_create1/5(in5)
  octal_mux0/6(out5) -> bus_create1/6(in6)
  octal_mux0/7(out6) -> bus_create1/7(in7)
  octal_mux0/8(out7) -> bus_create1/8(in8)
  Delay/1 -> bus_create5/1(in1)
  Delay1/1 -> bus_create5/2(in2)
  Delay2/1 -> bus_create5/3(in3)
  Delay3/1 -> bus_create5/4(in4)
  Delay4/1 -> bus_create5/5(in5)
  Delay5/1 -> bus_create5/6(in6)
  Delay6/1 -> bus_create5/7(in7)
  Delay7/1 -> bus_create5/8(in8)
  octal_mux1/8(out7) -> bus_create2/8(in8)
  octal_mux1/7(out6) -> bus_create2/7(in7)
  octal_mux1/6(out5) -> bus_create2/6(in6)
  octal_mux1/5(out4) -> bus_create2/5(in5)
  octal_mux1/4(out3) -> bus_create2/4(in4)
  octal_mux1/3(out2) -> bus_create2/3(in3)
  octal_mux1/2(out1) -> bus_create2/2(in2)
  octal_mux1/1(out0) -> bus_create2/1(in1)
  [noise7] -> octal_mux1/8(n7)
  [noise6] -> octal_mux1/7(n6)
  [noise5] -> octal_mux1/6(n5)
  [noise4] -> octal_mux1/5(n4)
  [noise3] -> octal_mux1/4(n3)
  [noise2] -> octal_mux1/3(n2)
  [noise1] -> octal_mux1/2(n1)
  [noise0] -> octal_mux1/1(n0)
  [noise0] -> octal_mux2/1(n0)
  [noise1] -> octal_mux2/2(n1)
  [noise2] -> octal_mux2/3(n2)
  [noise3] -> octal_mux2/4(n3)
  [noise4] -> octal_mux2/5(n4)
  [noise5] -> octal_mux2/6(n5)
  [noise6] -> octal_mux2/7(n6)
  [noise7] -> octal_mux2/8(n7)
  octal_mux2/1(out0) -> bus_create3/1(in1)
  octal_mux2/2(out1) -> bus_create3/2(in2)
  octal_mux2/3(out2) -> bus_create3/3(in3)
  octal_mux2/4(out3) -> bus_create3/4(in4)
  octal_mux2/5(out4) -> bus_create3/5(in5)
  octal_mux2/6(out5) -> bus_create3/6(in6)
  octal_mux2/7(out6) -> bus_create3/7(in7)
  octal_mux2/8(out7) -> bus_create3/8(in8)
  octal_mux3/8(out7) -> bus_create4/8(in8)
  octal_mux3/7(out6) -> bus_create4/7(in7)
  octal_mux3/6(out5) -> bus_create4/6(in6)
  octal_mux3/5(out4) -> bus_create4/5(in5)
  octal_mux3/4(out3) -> bus_create4/4(in4)
  octal_mux3/3(out2) -> bus_create4/3(in3)
  octal_mux3/2(out1) -> bus_create4/2(in2)
  octal_mux3/1(out0) -> bus_create4/1(in1)
  [noise7] -> octal_mux3/8(n7)
  [noise6] -> octal_mux3/7(n6)
  [noise5] -> octal_mux3/6(n5)
  [noise4] -> octal_mux3/5(n4)
  [noise3] -> octal_mux3/4(n3)
  [noise2] -> octal_mux3/3(n2)
  [noise1] -> octal_mux3/2(n1)
  [noise0] -> octal_mux3/1(n0)
  [noise0] -> octal_mux7/1(n0)
  [noise1] -> octal_mux7/2(n1)
  [noise2] -> octal_mux7/3(n2)
  [noise3] -> octal_mux7/4(n3)
  [noise4] -> octal_mux7/5(n4)
  [noise5] -> octal_mux7/6(n5)
  [noise6] -> octal_mux7/7(n6)
  [noise7] -> octal_mux7/8(n7)
  octal_mux7/1(out0) -> bus_create9/1(in1)
  octal_mux7/2(out1) -> bus_create9/2(in2)
  octal_mux7/3(out2) -> bus_create9/3(in3)
  octal_mux7/4(out3) -> bus_create9/4(in4)
  octal_mux7/5(out4) -> bus_create9/5(in5)
  octal_mux7/6(out5) -> bus_create9/6(in6)
  octal_mux7/7(out6) -> bus_create9/7(in7)
  octal_mux7/8(out7) -> bus_create9/8(in8)
  octal_mux6/8(out7) -> bus_create8/8(in8)
  octal_mux6/7(out6) -> bus_create8/7(in7)
  octal_mux6/6(out5) -> bus_create8/6(in6)
  octal_mux6/5(out4) -> bus_create8/5(in5)
  octal_mux6/4(out3) -> bus_create8/4(in4)
  octal_mux6/3(out2) -> bus_create8/3(in3)
  octal_mux6/2(out1) -> bus_create8/2(in2)
  octal_mux6/1(out0) -> bus_create8/1(in1)
  [noise7] -> octal_mux6/8(n7)
  [noise6] -> octal_mux6/7(n6)
  [noise5] -> octal_mux6/6(n5)
  [noise4] -> octal_mux6/5(n4)
  [noise3] -> octal_mux6/4(n3)
  [noise2] -> octal_mux6/3(n2)
  [noise1] -> octal_mux6/2(n1)
  [noise0] -> octal_mux6/1(n0)
  [noise0] -> octal_mux5/1(n0)
  [noise1] -> octal_mux5/2(n1)
  [noise2] -> octal_mux5/3(n2)
  [noise3] -> octal_mux5/4(n3)
  [noise4] -> octal_mux5/5(n4)
  [noise5] -> octal_mux5/6(n5)
  [noise6] -> octal_mux5/7(n6)
  [noise7] -> octal_mux5/8(n7)
  octal_mux5/1(out0) -> bus_create7/1(in1)
  octal_mux5/2(out1) -> bus_create7/2(in2)
  octal_mux5/3(out2) -> bus_create7/3(in3)
  octal_mux5/4(out3) -> bus_create7/4(in4)
  octal_mux5/5(out4) -> bus_create7/5(in5)
  octal_mux5/6(out5) -> bus_create7/6(in6)
  octal_mux5/7(out6) -> bus_create7/7(in7)
  octal_mux5/8(out7) -> bus_create7/8(in8)
  octal_mux4/8(out7) -> bus_create6/8(in8)
  octal_mux4/7(out6) -> bus_create6/7(in7)
  octal_mux4/6(out5) -> bus_create6/6(in6)
  octal_mux4/5(out4) -> bus_create6/5(in5)
  octal_mux4/4(out3) -> bus_create6/4(in4)
  octal_mux4/3(out2) -> bus_create6/3(in3)
  octal_mux4/2(out1) -> bus_create6/2(in2)
  octal_mux4/1(out0) -> bus_create6/1(in1)
  [noise7] -> octal_mux4/8(n7)
  [noise6] -> octal_mux4/7(n6)
  [noise5] -> octal_mux4/6(n5)
  [noise4] -> octal_mux4/5(n4)
  [noise3] -> octal_mux4/4(n3)
  [noise2] -> octal_mux4/3(n2)
  [noise1] -> octal_mux4/2(n1)
  [noise0] -> octal_mux4/1(n0)
  bus_create1/1(bus_out) -> Delay/1
  bus_create2/1(bus_out) -> Delay1/1
  bus_create3/1(bus_out) -> Delay2/1
  bus_create4/1(bus_out) -> Delay3/1
  bus_create6/1(bus_out) -> Delay4/1
  bus_create7/1(bus_out) -> Delay5/1
  bus_create8/1(bus_out) -> Delay6/1
  bus_create9/1(bus_out) -> Delay7/1
